simulink model "SdbRxSigProc"  (R2022b) (continued)
== system SdbRxSigProc/SdbRxSigProc_Runnable_sys == (continued)
  DrvrSeatVentnDesPwrCns2_DrvrSeatVentnDesPwrCns2_read/1 -> Terminator_151/1
  DrvrSeatVentnLvlSts2_DrvrSeatVentnLvlSts2_read/1 -> Terminator_152/1
  EngSt1WdSts_EngSt1WdSts_read/1 -> Terminator_153/1
  EngSt1WdSts_EngSt1WdSts_IsUpdated_read/1 -> Terminator_154/1
  FctvSts_FctvSts_read/1 -> Terminator_155/1
  FloatDrvingActv_FloatDrvingActv_read/1 -> Terminator_156/1
  FrntAirPtcHVPwrActl_FrntAirPtcHVPwrActl_read/1 -> Terminator_157/1
  FrntAirPtcHVPwrActl_FrntAirPtcHVPwrActl_IsUpdated_read/1 -> Terminator_158/1
  HighTempCleanHmiReq_HighTempCleanHmiReq_read/1 -> Terminator_159/1
  HmiAC_HmiAC_read/1 -> Terminator_160/1
  HmiAC_HmiAC_IsUpdated_read/1 -> Terminator_161/1
  HMIAirCdnrAutDrySet_HMIAirCdnrAutDrySet_read/1 -> Terminator_162/1
  HMIAirCdnrAutDrySet_HMIAirCdnrAutDrySet_IsUpdated_read/1 -> Terminator_163/1
  HmiAirDistbnRow1_HmiAirDistbnRow1_read/1 -> Terminator_164/1
  HmiAirDistbnRow1_HmiAirDistbnRow1_IsUpdated_read/1 -> Terminator_165/1
  HmiAirDistbnRow1Le_HmiAirDistbnRow1Le_read/1 -> Terminator_166/1
  HmiAirDistbnRow1Ri_HmiAirDistbnRow1Ri_read/1 -> Terminator_167/1
  HmiAirDistbnRow2_HmiAirDistbnRow2_read/1 -> Terminator_168/1
  HmiAirDistbnRow2_HmiAirDistbnRow2_IsUpdated_read/1 -> Terminator_169/1
  HmiAutoRow1_HmiAutoRow1_read/1 -> Terminator_170/1
  HmiAutoRow1_HmiAutoRow1_IsUpdated_read/1 -> Terminator_171/1
  HmiAutoRow1Le_HmiAutoRow1Le_read/1 -> Terminator_172/1
  HmiAutoRow1Ri_HmiAutoRow1Ri_read/1 -> Terminator_173/1
  HmiAutoRow2_HmiAutoRow2_read/1 -> Terminator_174/1
  HmiAutoRow2_HmiAutoRow2_IsUpdated_read/1 -> Terminator_175/1
  HmiAutOxySet_HmiAutOxySet_read/1 -> Terminator_176/1
  HMIBluetoothCallToAdjAirVol_HMIBluetoothCallToAdjAirVol_read/1 -> Terminator_177/1
  HmiCarLockACRecircSet_HmiCarLockACRecircSet_read/1 -> Terminator_178/1
  HmiCbnOverHeatPrevnReq_HmiCbnOverHeatPrevnReq_read/1 -> Terminator_179/1
  HmiCbnOverHeatPrevnReq_HmiCbnOverHeatPrevnReq_IsUpdated_read/1 -> Terminator_180/1
  HmiClimaRow1LePwr_HmiClimaRow1LePwr_read/1 -> Terminator_181/1
  HmiClimaRow1Pwr_HmiClimaRow1Pwr_read/1 -> Terminator_182/1
  HmiClimaRow1RiPwr_HmiClimaRow1RiPwr_read/1 -> Terminator_183/1
  HmiClimaRow2Pwr_HmiClimaRow2Pwr_read/1 -> Terminator_184/1
  HmiClimaRow3Pwr_HmiClimaRow3Pwr_read/1 -> Terminator_185/1
  HMIClimaVentSet_HMIClimaVentSet_read/1 -> Terminator_186/1
  HmiCmptmtAirDistbnFrnt_HmiCmptmtAirDistbnFrnt_read/1 -> Terminator_187/1
  HmiDrvrHeadPosn_HmiDrvrHeadPosn_read/1 -> Terminator_188/1
  HmiEcoClimaReq_HmiEcoClimaReq_read/1 -> Terminator_189/1
  HmiEcoClimaReq_HmiEcoClimaReq_IsUpdated_read/1 -> Terminator_190/1
  HmiElecAirBodyVentLebtn_HmiElecAirBodyVentLebtn_read/1 -> Terminator_191/1
  HmiElecAirBodyVentRibtn_HmiElecAirBodyVentRibtn_read/1 -> Terminator_192/1
  HmiElecAirConsolePosn_HmiElecAirConsolePosn_read/1 -> Terminator_193/1
  HmiElecAirConsoleSwtLe_HmiElecAirConsoleSwtLe_read/1 -> Terminator_194/1
  HmiElecAirConsoleSwtRi_HmiElecAirConsoleSwtRi_read/1 -> Terminator_195/1
  HmiElecAirDirModeSecRowReq_HmiElecAirDirModeSecRowReq_read/1 -> Terminator_196/1
  HmiElecAirDirSwngSecLe_HmiElecAirDirSwngSecLe_read/1 -> Terminator_197/1
  HmiElecAirDirSwngSecRi_HmiElecAirDirSwngSecRi_read/1 -> Terminator_198/1
  HmiElecAirDrvrNatureWindReq_HmiElecAirDrvrNatureWindReq_read/1 -> Terminator_199/1
  HmiElecAirPassNatureWindReq_HmiElecAirPassNatureWindReq_read/1 -> Terminator_200/1
  HMIFACSwt_HMIFACSwt_read/1 -> Terminator_201/1
  HmiFanLvlRow1_HmiFanLvlRow1_read/1 -> Terminator_202/1
  HmiFanLvlRow1_HmiFanLvlRow1_IsUpdated_read/1 -> Terminator_203/1
  HmiFanLvlRow1Le_HmiFanLvlRow1Le_read/1 -> Terminator_204/1
  HmiFanLvlRow1Ri_HmiFanLvlRow1Ri_read/1 -> Terminator_205/1
  HmiFanLvlRow2_HmiFanLvlRow2_read/1 -> Terminator_206/1
  HmiFanLvlRow2_HmiFanLvlRow2_IsUpdated_read/1 -> Terminator_207/1
  HmiFragraCh_HmiFragraCh_read/1 -> Terminator_208/1
  HmiFragraCh_HmiFragraCh_IsUpdated_read/1 -> Terminator_209/1
  HmiFragraLvl_HmiFragraLvl_read/1 -> Terminator_210/1
  HmiFragraLvl_HmiFragraLvl_IsUpdated_read/1 -> Terminator_211/1
  HMIIonizerAirCdnr_HMIIonizerAirCdnr_read/1 -> Terminator_212/1
  HMIIonizerAirCdnr_HMIIonizerAirCdnr_IsUpdated_read/1 -> Terminator_213/1
  HMILBRACStrtSet_HMILBRACStrtSet_read/1 -> Terminator_214/1
  HmiMaxAC_HmiMaxAC_read/1 -> Terminator_215/1
  HmiMaxAC_HmiMaxAC_IsUpdated_read/1 -> Terminator_216/1
  HmiMaxDefrst_HmiMaxDefrst_read/1 -> Terminator_217/1
  HmiMaxDefrst_HmiMaxDefrst_IsUpdated_read/1 -> Terminator_218/1
  HmiOxyinCarSwt_HmiOxyinCarSwt_read/1 -> Terminator_219/1
  HmiParkVentSet_HmiParkVentSet_read/1 -> Terminator_220/1
  HmiParkVentTmrSet_HmiParkVentTmrSet_read/1 -> Terminator_221/1
  HmiPassHeadPosn_HmiPassHeadPosn_read/1 -> Terminator_222/1
  HmiPM25PopUpResp_HmiPM25PopUpResp_read/1 -> Terminator_223/1
  HmiPM25PopUpResp_HmiPM25PopUpResp_IsUpdated_read/1 -> Terminator_224/1
  HmiPM25PopUpSet_HmiPM25PopUpSet_read/1 -> Terminator_225/1
  HmiPM25PopUpSet_HmiPM25PopUpSet_IsUpdated_read/1 -> Terminator_226/1
  HmiSmrtWindFolwSet_HmiSmrtWindFolwSet_read/1 -> Terminator_227/1
  HMIThermoTankDoorOpen_HMIThermoTankDoorOpen_read/1 -> Terminator_228/1
  HMIThermoTankEcoSw_HMIThermoTankEcoSw_read/1 -> Terminator_229/1
  HMIThermoTankT_HMIThermoTankT_read/1 -> Terminator_230/1
  HmiThermoTSteDeoNewSwt_HmiThermoTSteDeoNewSwt_read/1 -> Terminator_231/1
  HmiThermoTSteDeoSwt_HmiThermoTSteDeoSwt_read/1 -> Terminator_232/1
  HmiTSyncn_HmiTSyncn_read/1 -> Terminator_233/1
  HmiTSyncn_HmiTSyncn_IsUpdated_read/1 -> Terminator_234/1
  HmiUVSterilSet_HmiUVSterilSet_read/1 -> Terminator_235/1
  HmiWinterLockCarPrevnIceSet_HmiWinterLockCarPrevnIceSet_read/1 -> Terminator_236/1
  HmiWinterLockCarPrevnIceSet_HmiWinterLockCarPrevnIceSet_IsUpdated_read/1 -> Terminator_237/1
  HoodSts_HoodSts_read/1 -> Terminator_238/1
  HvBattLimnIndcn_HvBattLimnIndcn_read/1 -> Terminator_239/1
  InflateAirSrcSwt_InflateAirSrcSwt_read/1 -> Terminator_240/1
  InpVoltFb_InpVoltFb_read/1 -> Terminator_241/1
  LiOprnMod_LiOprnMod_read/1 -> Terminator_242/1
  LonglifeBatteryReq_LonglifeBatteryReq_read/1 -> Terminator_243/1
  McuOvrTempErr_McuOvrTempErr_read/1 -> Terminator_244/1
  McuTempFb_McuTempFb_read/1 -> Terminator_245/1
  McuTempSnsrErr_McuTempSnsrErr_read/1 -> Terminator_246/1
  MoleculeVlv1_MoleculeVlv1_read/1 -> Terminator_247/1
  MoleculeVlv2_MoleculeVlv2_read/1 -> Terminator_248/1
  MosOvrTempErr_MosOvrTempErr_read/1 -> Terminator_249/1
  MosTempFb_MosTempFb_read/1 -> Terminator_250/1
  MosTempSnsrErr_MosTempSnsrErr_read/1 -> Terminator_251/1
  MotStsFrmOGM_MotStsFrmOGM_read/1 -> Terminator_252/1
  MotTempFb_MotTempFb_read/1 -> Terminator_253/1
  MotTempSnsrErr_MotTempSnsrErr_read/1 -> Terminator_254/1
  OGMCmprSpdFb_OGMCmprSpdFb_read/1 -> Terminator_255/1
  OGMFlowFb_OGMFlowFb_read/1 -> Terminator_256/1
  OGMHwVers_OGMHwVers_read/1 -> Terminator_257/1
  OGMIntErr_OGMIntErr_read/1 -> Terminator_258/1
  OGMOvrVoltRangErr_OGMOvrVoltRangErr_read/1 -> Terminator_259/1
  OGMRunTi_OGMRunTi_read/1 -> Terminator_260/1
  OGMSwVers_OGMSwVers_read/1 -> Terminator_261/1
  OGMTempErr_OGMTempErr_read/1 -> Terminator_262/1
  OGMUndVoltRangErr_OGMUndVoltRangErr_read/1 -> Terminator_263/1
  OxygenConcFbFrmOGM_OxygenConcFbFrmOGM_read/1 -> Terminator_264/1
  OxygenConcFlt_OxygenConcFlt_read/1 -> Terminator_265/1
  OxygenConcSnsrElecErr_OxygenConcSnsrElecErr_read/1 -> Terminator_266/1
  OxygenConcSnsrIntErr_OxygenConcSnsrIntErr_read/1 -> Terminator_267/1
  OxygenConcSnsrOvrVoltRangErr_OxygenConcSnsrOvrVoltRangErr_read/1 -> Terminator_268/1
  OxygenConcSnsrSts_OxygenConcSnsrSts_read/1 -> Terminator_269/1
  OxygenConcSnsrStsFrmOGM_OxygenConcSnsrStsFrmOGM_read/1 -> Terminator_270/1
  OxygenConcSnsrTempErr_OxygenConcSnsrTempErr_read/1 -> Terminator_271/1
  OxygenConcSnsrUndVoltRangErr_OxygenConcSnsrUndVoltRangErr_read/1 -> Terminator_272/1
  OxygenGenrErrInfo_OxygenGenrErrInfo_read/1 -> Terminator_273/1
  OxygenSplyAvlSts_OxygenSplyAvlSts_read/1 -> Terminator_274/1
  OxygenSplyErr_OxygenSplyErr_read/1 -> Terminator_275/1
  OxygenTubeBlk_OxygenTubeBlk_read/1 -> Terminator_276/1
  PassSeatPosPerc_PassSeatPosPerc_read/1 -> Terminator_277/1
  PassSeatPosPerc_PassSeatPosPerc_IsUpdated_read/1 -> Terminator_278/1
  PassSeatSts2_PassSeatSts2_read/1 -> Terminator_279/1
  PassSeatVentnActlPwrCns2_PassSeatVentnActlPwrCns2_read/1 -> Terminator_280/1
  PassSeatVentnDesPwrCns2_PassSeatVentnDesPwrCns2_read/1 -> Terminator_281/1
  PassSeatVentnLvlSts2_PassSeatVentnLvlSts2_read/1 -> Terminator_282/1
  PhaseCurrentFb_PhaseCurrentFb_read/1 -> Terminator_283/1
  PosnBrief_PosnBrief_read/1 -> Terminator_284/1
  PressureFb_PressureFb_read/1 -> Terminator_285/1
  PressureFlt_PressureFlt_read/1 -> Terminator_286/1
  PressureSnsrErr_PressureSnsrErr_read/1 -> Terminator_287/1
  RefrLpSwSts_RefrLpSwSts_read/1 -> Terminator_288/1
  RefrtorVlvEna_RefrtorVlvEna_read/1 -> Terminator_289/1
  ResrvdSigForCCM2_ResrvdSigForCCM2_read/1 -> Terminator_290/1
  RoadIncln_RoadIncln_read/1 -> Terminator_291/1
  RuranACModeSet_RuranACModeSet_read/1 -> Terminator_292/1
  SeatBackAngleRowFirstDrvr_SeatBackAngleRowFirstDrvr_read/1 -> Terminator_293/1
  SeatBackAngleRowFirstDrvr_SeatBackAngleRowFirstDrvr_IsUpdated_read/1 -> Terminator_294/1
  SeatBackAngleRowFirstPass_SeatBackAngleRowFirstPass_read/1 -> Terminator_295/1
  SeatBackAngleRowFirstPass_SeatBackAngleRowFirstPass_IsUpdated_read/1 -> Terminator_296/1
  SeatOccptAtRowSecDrSide2_SeatOccptAtRowSecDrSide2_read/1 -> Terminator_297/1
  SeatOccptAtRowSecMid2_SeatOccptAtRowSecMid2_read/1 -> Terminator_298/1
  SeatOccptAtRowSecPasSide2_SeatOccptAtRowSecPasSide2_read/1 -> Terminator_299/1
  SeatOccptAtRowThrdLe2_SeatOccptAtRowThrdLe2_read/1 -> Terminator_300/1
  SeatOccptAtRowThrdRi2_SeatOccptAtRowThrdRi2_read/1 -> Terminator_301/1
  SportModeThmgtRequest_SportModeThmgtRequest_read/1 -> Terminator_302/1
  TCSCooltFlow_TCSCooltFlow_read/1 -> Terminator_303/1
  TCSCooltT_TCSCooltT_read/1 -> Terminator_304/1
  TcsDoorEleBailReq_TcsDoorEleBailReq_read/1 -> Terminator_305/1
  TcsFanReq_TcsFanReq_read/1 -> Terminator_306/1
  TcsModSwtReq_TcsModSwtReq_read/1 -> Terminator_307/1
  TcsOvrheatdProtnSts_TcsOvrheatdProtnSts_read/1 -> Terminator_308/1
  TcsSemiconReq_TcsSemiconReq_read/1 -> Terminator_309/1
  TcsTmrOverReq_TcsTmrOverReq_read/1 -> Terminator_310/1
  TcsTNowCtrlReq_TcsTNowCtrlReq_read/1 -> Terminator_311/1
  TcsTopDoorEleBailReq_TcsTopDoorEleBailReq_read/1 -> Terminator_312/1
  TelmOxygenValReq_TelmOxygenValReq_read/1 -> Terminator_313/1
  TelmThermoTankECOSet_TelmThermoTankECOSet_read/1 -> Terminator_314/1
  TelmThermoTankT_TelmThermoTankT_read/1 -> Terminator_315/1
  ThermHvacRecircReq_ThermHvacRecircReq_read/1 -> Terminator_316/1
  ThermHvacRecircReqEna_ThermHvacRecircReqEna_read/1 -> Terminator_317/1
  ThrdRowSeatHeatgActlPwrCnsLe_ThrdRowSeatHeatgActlPwrCnsLe_read/1 -> Terminator_318/1
  ThrdRowSeatHeatgActlPwrCnsRi_ThrdRowSeatHeatgActlPwrCnsRi_read/1 -> Terminator_319/1
  ThrdRowSeatHeatgDesPwrCnsLe_ThrdRowSeatHeatgDesPwrCnsLe_read/1 -> Terminator_320/1
  ThrdRowSeatHeatgDesPwrCnsRi_ThrdRowSeatHeatgDesPwrCnsRi_read/1 -> Terminator_321/1
  ThrdRowSeatVentnActlPwrCnsLe_ThrdRowSeatVentnActlPwrCnsLe_read/1 -> Terminator_322/1
  ThrdRowSeatVentnActlPwrCnsRi_ThrdRowSeatVentnActlPwrCnsRi_read/1 -> Terminator_323/1
  ThrdRowSeatVentnDesPwrCnsLe_ThrdRowSeatVentnDesPwrCnsLe_read/1 -> Terminator_324/1
  ThrdRowSeatVentnDesPwrCnsRi_ThrdRowSeatVentnDesPwrCnsRi_read/1 -> Terminator_325/1
  UseEvapReqTMinNew_UseEvapReqTMinNew_read/1 -> Terminator_326/1
  VlvVoltFlt_VlvVoltFlt_read/1 -> Terminator_327/1
  WarnLvlMsg_WarnLvlMsg_read/1 -> Terminator_328/1
  WarnLvlMsg_WarnLvlMsg_IsUpdated_read/1 -> Terminator_329/1
  WasRespLevel_WasRespLevel_read/1 -> Terminator_330/1
  WasRespLevel_WasRespLevel_IsUpdated_read/1 -> Terminator_331/1
  WaterDepthInformation_WaterDepthInformation_read/1 -> Terminator_332/1
  WeatherQualityAQI_WeatherQualityAQI_read/1 -> Terminator_333/1
  Ground_1/1 -> AACAirClngErrStsNgcc_AACAirClngErrStsNgcc_write/1
  Ground_2/1 -> AACAirClngStsNgcc_AACAirClngStsNgcc_write/1
  Ground_3/1 -> AFUFragraCh1AvlTiPrecNgcc_AFUFragraCh1AvlTiPrecNgcc_write/1
  Ground_4/1 -> AFUFragraCh2AvlTiPrecNgcc_AFUFragraCh2AvlTiPrecNgcc_write/1
  Ground_5/1 -> AFUFragraCh3AvlTiPrecNgcc_AFUFragraCh3AvlTiPrecNgcc_write/1
  Ground_6/1 -> AFUFragraCh4AvlTiPrecNgcc_AFUFragraCh4AvlTiPrecNgcc_write/1
  Ground_7/1 -> AFUFragraCh5AvlTiPrecNgcc_AFUFragraCh5AvlTiPrecNgcc_write/1
  Ground_8/1 -> AirClngReq_AirClngReq_write/1
  Ground_9/1(Out1) -> AirFragCh1OvrdDyNgcc_AirFragCh1OvrdDyNgcc_write/1
  Ground_10/1(Out1) -> AirFragCh2OvrdDyNgcc_AirFragCh2OvrdDyNgcc_write/1
  Ground_11/1(Out1) -> AirFragCh3OvrdDyNgcc_AirFragCh3OvrdDyNgcc_write/1
  Ground_12/1(Out1) -> AirFragCh4OvrdDyNgcc_AirFragCh4OvrdDyNgcc_write/1
  Ground_13/1(Out1) -> AirFragCh5OvrdDyNgcc_AirFragCh5OvrdDyNgcc_write/1
  Ground_14/1 -> AirPtcEnadNgcc_AirPtcEnadNgcc_write/1
  Ground_15/1 -> AirPtcHVPwrActlFirstLeNgcc_AirPtcHVPwrActlFirstLeNgcc_write/1
  Ground_16/1 -> AirPtcHVPwrActlFirstRiNgcc_AirPtcHVPwrActlFirstRiNgcc_write/1
  Ground_17/1 -> AirPtcHVPwrActlSecLeNgcc_AirPtcHVPwrActlSecLeNgcc_write/1
  Ground_18/1 -> AirPtcModeReqNgcc_AirPtcModeReqNgcc_write/1
  Ground_19/1 -> AirSrcStsFbNgcc_AirSrcStsFbNgcc_write/1
  Ground_20/1 -> AltitudeInfromNgcc_AltitudeInfromNgcc_write/1
  Ground_21/1(Out1) -> AMBStsNgcc_AMBStsNgcc_write/1
  Ground_22/1 -> BackflushVlvErrNgcc_BackflushVlvErrNgcc_write/1
  Ground_23/1 -> BookTrvlClimaActvReq_Ngcc_BookTrvlClimaActvReq_write/1
  Ground_24/1 -> BusCurrentFbNgcc_BusCurrentFbNgcc_write/1
  Ground_25/1 -> ChgFilElmWarnNgcc_ChgFilElmWarnNgcc_write/1
  Ground_26/1 -> CmptmtOxygenConcQFNgcc_CmptmtOxygenConcQFNgcc_write/1
  Ground_27/1 -> CmptmtOxygenConcVluNgcc_CmptmtOxygenConcVluNgcc_write/1
  Ground_28/1 -> CoolgFanErrNgcc_CoolgFanErrNgcc_write/1
  Ground_29/1(Out1) -> CourseDirFromNavNgcc_CourseDirFromNavNgcc_write/1
  Ground_30/1 -> CurrentCongestionInformationNgcc_CurrentCongestionInformationNgcc_write/1
  Ground_31/1 -> CurrentTunnelInformationNgcc_CurrentTunnelInformationNgcc_write/1
  Ground_32/1 -> DcDcActvdNgcc_DcDcActvdNgcc_write/1
  Ground_33/1 -> DcDcPwrLimNgcc_DcDcPwrLimNgcc_write/1
  Ground_34/1 -> DHUResdSig10Ngcc_DHUResdSig10Ngcc_write/1
  Ground_35/1 -> DHUResdSig11Ngcc_DHUResdSig11Ngcc_write/1
  Ground_36/1 -> DHUResdSig12Ngcc_DHUResdSig12Ngcc_write/1
  Ground_37/1 -> DHUResdSig13Ngcc_DHUResdSig13Ngcc_write/1
  Ground_38/1 -> DHUResdSig3Ngcc_DHUResdSig3Ngcc_write/1
  Ground_39/1 -> DHUResdSig4Ngcc_DHUResdSig4Ngcc_write/1
  Ground_40/1 -> DHUResdSig5Ngcc_DHUResdSig5Ngcc_write/1
  Ground_41/1 -> DHUResdSig6Ngcc_DHUResdSig6Ngcc_write/1
  Ground_42/1 -> DHUResdSig7Ngcc_DHUResdSig7Ngcc_write/1
  Ground_43/1 -> DHUResdSig8Ngcc_DHUResdSig8Ngcc_write/1
  Ground_44/1 -> DHUResdSig9Ngcc_DHUResdSig9Ngcc_write/1
  Ground_45/1 -> DispHvBattLvlOfChrgNgcc_DispHvBattLvlOfChrgNgcc_write/1
  Ground_46/1 -> DropTopPosnStsNgcc_DropTopPosnStsNgcc_write/1
  Ground_47/1(Out1) -> DrvrSeatPosPercNgcc_DrvrSeatPosPercNgcc_write/1
  Ground_48/1 -> DrvrSeatSts_Ngcc_DrvrSeatSts_write/1
  Ground_49/1 -> DrvrSeatVentnLvlSts2Ngcc_DrvrSeatVentnLvlSts2Ngcc_write/1
  Ground_50/1(Out1) -> FctvStsNgcc_FctvStsNgcc_write/1
  Ground_51/1(Out1) -> FloatDrvingActvNgcc_FloatDrvingActvNgcc_write/1
  Ground_52/1 -> HighTempCleanHmiReqNgcc_HighTempCleanHmiReqNgcc_write/1
  Ground_53/1 -> HMIAirCdnrAutDrySetNgcc_HMIAirCdnrAutDrySetNgcc_write/1
  Ground_54/1 -> HmiAirDistbnRow1LeNgcc_HmiAirDistbnRow1LeNgcc_write/1
  Ground_55/1 -> HmiAirDistbnRow1RiNgcc_HmiAirDistbnRow1RiNgcc_write/1
  Ground_56/1 -> HmiAutoRow1LeNgcc_HmiAutoRow1LeNgcc_write/1
  Ground_57/1 -> HmiAutoRow1RiNgcc_HmiAutoRow1RiNgcc_write/1
  Ground_58/1 -> HmiAutOxySetNgcc_HmiAutOxySetNgcc_write/1
  Ground_59/1 -> HmiCarLockACRecircSetNgcc_HmiCarLockACRecircSetNgcc_write/1
  Ground_60/1 -> HmiClimaRow1LePwrNgcc_HmiClimaRow1LePwrNgcc_write/1
  Ground_61/1 -> HmiClimaRow1PwrNgcc_HmiClimaRow1PwrNgcc_write/1
  Ground_62/1 -> HmiClimaRow1RiPwrNgcc_HmiClimaRow1RiPwrNgcc_write/1
  Ground_63/1 -> HmiClimaRow2PwrNgcc_HmiClimaRow2PwrNgcc_write/1
  Ground_64/1 -> HmiClimaRow3PwrNgcc_HmiClimaRow3PwrNgcc_write/1
  Ground_65/1 -> HMIClimaVentSetNgcc_HMIClimaVentSetNgcc_write/1
  Ground_66/1(Out1) -> HmiDrvrHeadPosnNgcc_HmiDrvrHeadPosnNgcc_write/1
  Ground_67/1 -> HmiElecAirBodyVentLebtnNgcc_HmiElecAirBodyVentLebtnNgcc_write/1
  Ground_68/1 -> HmiElecAirBodyVentRibtnNgcc_HmiElecAirBodyVentRibtnNgcc_write/1
  Ground_69/1(Out1) -> HmiElecAirConsolePosnNgcc_HmiElecAirConsolePosnNgcc_write/1
  Ground_70/1 -> HmiElecAirConsoleSwtLeNgcc_HmiElecAirConsoleSwtLeNgcc_write/1
  Ground_71/1 -> HmiElecAirConsoleSwtRiNgcc_HmiElecAirConsoleSwtRiNgcc_write/1
  Ground_72/1(Out1) -> HmiElecAirDirModeSecRowReqNgcc_HmiElecAirDirModeSecRowReqNgcc_write/1
  Ground_73/1 -> HmiElecAirDirSwngSecLeNgcc_HmiElecAirDirSwngSecLeNgcc_write/1
  Ground_74/1 -> HmiElecAirDirSwngSecRiNgcc_HmiElecAirDirSwngSecRiNgcc_write/1
  Ground_75/1 -> HmiElecAirDrvrNatureWindReqNgcc_HmiElecAirDrvrNatureWindReqNgcc_write/1
  Ground_76/1 -> HmiElecAirPassNatureWindReqNgcc_HmiElecAirPassNatureWindReqNgcc_write/1
  Ground_77/1 -> HMIFACSwtNgcc_HMIFACSwtNgcc_write/1
  Ground_78/1 -> HmiFanLvlRow1LeNgcc_HmiFanLvlRow1LeNgcc_write/1
  Ground_79/1 -> HmiFanLvlRow1RiNgcc_HmiFanLvlRow1RiNgcc_write/1
  Ground_80/1(Out1) -> HmiFragraChRatReq_HmiFragraChRatReq_write/1
  Ground_81/1 -> HmiFragraLvlReq_HmiFragraLvlReq_write/1
  Ground_82/1 -> HMILBRACStrtSetNgcc_HMILBRACStrtSetNgcc_write/1
  Ground_83/1 -> HmiOxyinCarSwtNgcc_HmiOxyinCarSwtNgcc_write/1
  Ground_84/1 -> HmiParkVentSetNgcc_HmiParkVentSetNgcc_write/1
  Ground_85/1 -> HmiParkVentTmrSetNgcc_HmiParkVentTmrSetNgcc_write/1
  Ground_86/1(Out1) -> HmiPassHeadPosnNgcc_HmiPassHeadPosnNgcc_write/1
  Ground_87/1 -> HmiPopUpResp_HmiPopUpResp_write/1
  Ground_88/1 -> HmiSmrtWindFolwSetNgcc_HmiSmrtWindFolwSetNgcc_write/1
  Ground_89/1 -> HMIThermoTankDoorOpenNgcc_HMIThermoTankDoorOpenNgcc_write/1
  Ground_90/1 -> HMIThermoTankEcoSwNgcc_HMIThermoTankEcoSwNgcc_write/1
  Ground_91/1 -> HMIThermoTankTNgcc_HMIThermoTankTNgcc_write/1
  Ground_92/1 -> HmiThermoTSteDeoNewSwtNgcc_HmiThermoTSteDeoNewSwtNgcc_write/1
  Ground_93/1 -> HmiThermoTSteDeoSwtNgcc_HmiThermoTSteDeoSwtNgcc_write/1
  Ground_94/1 -> HmiUVSterilSetNgcc_HmiUVSterilSetNgcc_write/1
  Ground_95/1 -> HmiWinterLockCarPrevnIceSetNgcc_HmiWinterLockCarPrevnIceSetNgcc_write/1
  Ground_96/1 -> HoodStsNgcc_HoodStsNgcc_write/1
  Ground_97/1 -> HvBattLimnIndcnNgcc_HvBattLimnIndcnNgcc_write/1
  Ground_98/1 -> InflateAirSrcSwtNgcc_InflateAirSrcSwtNgcc_write/1
  Ground_99/1 -> InpVoltFbNgcc_InpVoltFbNgcc_write/1
  Ground_100/1 -> LiOprnModNgcc_LiOprnModNgcc_write/1
  Ground_101/1 -> LonglifeBatteryReqNgcc_LonglifeBatteryReqNgcc_write/1
  Ground_102/1 -> McuOvrTempErrNgcc_McuOvrTempErrNgcc_write/1
  Ground_103/1 -> McuTempFbNgcc_McuTempFbNgcc_write/1
  Ground_104/1 -> McuTempSnsrErrNgcc_McuTempSnsrErrNgcc_write/1
  Ground_105/1 -> MoleculeVlv1Ngcc_MoleculeVlv1Ngcc_write/1
  Ground_106/1 -> MoleculeVlv2Ngcc_MoleculeVlv2Ngcc_write/1
  Ground_107/1 -> MosOvrTempErrNgcc_MosOvrTempErrNgcc_write/1
  Ground_108/1 -> MosTempFbNgcc_MosTempFbNgcc_write/1
  Ground_109/1 -> MosTempSnsrErrNgcc_MosTempSnsrErrNgcc_write/1
  Ground_110/1 -> MotStsFrmOGMNgcc_MotStsFrmOGMNgcc_write/1
  Ground_111/1 -> MotTempFbNgcc_MotTempFbNgcc_write/1
  Ground_112/1 -> MotTempSnsrErrNgcc_MotTempSnsrErrNgcc_write/1
  Ground_113/1 -> OGMCmprSpdFbNgcc_OGMCmprSpdFbNgcc_write/1
  Ground_114/1 -> OGMFlowFbNgcc_OGMFlowFbNgcc_write/1
  Ground_115/1 -> OGMHwVersNgcc_OGMHwVersNgcc_write/1
  Ground_116/1 -> OGMIntErrNgcc_OGMIntErrNgcc_write/1
  Ground_117/1 -> OGMOvrVoltRangErrNgcc_OGMOvrVoltRangErrNgcc_write/1
  Ground_118/1 -> OGMRunTiNgcc_OGMRunTiNgcc_write/1
  Ground_119/1 -> OGMSwVersNgcc_OGMSwVersNgcc_write/1
  Ground_120/1 -> OGMTempErrNgcc_OGMTempErrNgcc_write/1
  Ground_121/1 -> OGMUndVoltRangErrNgcc_OGMUndVoltRangErrNgcc_write/1
  Ground_122/1 -> OxygenConcFbFrmOGMNgcc_OxygenConcFbFrmOGMNgcc_write/1
  Ground_123/1 -> OxygenConcFltNgcc_OxygenConcFltNgcc_write/1
  Ground_124/1 -> OxygenConcSnsrElecErrNgcc_OxygenConcSnsrElecErrNgcc_write/1
  Ground_125/1 -> OxygenConcSnsrIntErrNgcc_OxygenConcSnsrIntErrNgcc_write/1
  Ground_126/1 -> OxygenConcSnsrOvrVoltRangErrNgcc_OxygenConcSnsrOvrVoltRangErrNgcc_write/1
  Ground_127/1 -> OxygenConcSnsrStsFrmOGMNgcc_OxygenConcSnsrStsFrmOGMNgcc_write/1
  Ground_128/1 -> OxygenConcSnsrStsNgcc_OxygenConcSnsrStsNgcc_write/1
  Ground_129/1 -> OxygenConcSnsrTempErrNgcc_OxygenConcSnsrTempErrNgcc_write/1
  Ground_130/1 -> OxygenConcSnsrUndVoltRangErrNgcc_OxygenConcSnsrUndVoltRangErrNgcc_write/1
  Ground_131/1 -> OxygenGenrErrInfoNgcc_OxygenGenrErrInfoNgcc_write/1
  Ground_132/1 -> OxygenSplyAvlStsNgcc_OxygenSplyAvlStsNgcc_write/1
  Ground_133/1 -> OxygenSplyErrNgcc_OxygenSplyErrNgcc_write/1
  Ground_134/1 -> OxygenTubeBlkNgcc_OxygenTubeBlkNgcc_write/1
  Ground_135/1(Out1) -> PassSeatPosPercNgcc_PassSeatPosPercNgcc_write/1
  Ground_136/1 -> PassSeatSts2_Ngcc_PassSeatSts2_write/1
  Ground_137/1 -> PassSeatVentnLvlSts2Ngcc_PassSeatVentnLvlSts2Ngcc_write/1
  Ground_138/1 -> PhaseCurrentFbNgcc_PhaseCurrentFbNgcc_write/1
  Ground_139/1(Out1) -> PosnBriefNgcc_PosnBriefNgcc_write/1
  Ground_140/1 -> PressureFbNgcc_PressureFbNgcc_write/1
  Ground_141/1 -> PressureFltNgcc_PressureFltNgcc_write/1
  Ground_142/1 -> PressureSnsrErrNgcc_PressureSnsrErrNgcc_write/1
  Ground_143/1 -> PwrCnsForHvacHeatrElecRe_PwrCnsForHvacHeatrElecRe_write/1
  Ground_144/1 -> RefrLpSwStsNgcc_RefrLpSwStsNgcc_write/1
  Ground_145/1 -> RefrtorVlvEnaNgcc_RefrtorVlvEnaNgcc_write/1
  Ground_146/1(Out1) -> RoadInclnNgcc_RoadInclnNgcc_write/1
  Ground_147/1 -> RuranACModeSetNgcc_RuranACModeSetNgcc_write/1
  Ground_148/1 -> SceneModSeld_Ngcc_SceneModSeld_write/1
  Ground_149/1 -> SeatBackAngleRowFirstDrvrNgcc_SeatBackAngleRowFirstDrvrNgcc_write/1
  Ground_150/1 -> SeatBackAngleRowFirstPassNgcc_SeatBackAngleRowFirstPassNgcc_write/1
  Ground_151/1 -> SeatOccptAtRowSecDrSide2_Ngcc_SeatOccptAtRowSecDrSide2_write/1
  Ground_152/1 -> SeatOccptAtRowSecMid2_Ngcc_SeatOccptAtRowSecMid2_write/1
  Ground_153/1 -> SeatOccptAtRowSecPasSide2_Ngcc_SeatOccptAtRowSecPasSide2_write/1
  Ground_154/1 -> SeatOccptAtRowThrdLe2Ngcc_SeatOccptAtRowThrdLe2Ngcc_write/1
  Ground_155/1 -> SeatOccptAtRowThrdRi2Ngcc_SeatOccptAtRowThrdRi2Ngcc_write/1
  Ground_156/1 -> SportModeThmgtRequestNgcc_SportModeThmgtRequestNgcc_write/1
  Ground_157/1 -> TCSCooltFlowNgcc_TCSCooltFlowNgcc_write/1
  Ground_158/1 -> TCSCooltTNgcc_TCSCooltTNgcc_write/1
  Ground_159/1 -> TcsDoorEleBailReqNgcc_TcsDoorEleBailReqNgcc_write/1
  Ground_160/1 -> TcsFanReqNgcc_TcsFanReqNgcc_write/1
  Ground_161/1 -> TcsModSwtReqNgcc_TcsModSwtReqNgcc_write/1
  Ground_162/1 -> TcsOvrheatdProtnStsNgcc_TcsOvrheatdProtnStsNgcc_write/1
  Ground_163/1 -> TcsSemiconReqNgcc_TcsSemiconReqNgcc_write/1
  Ground_164/1 -> TcsTmrOverReqNgcc_TcsTmrOverReqNgcc_write/1
  Ground_165/1 -> TcsTNowCtrlReqNgcc_TcsTNowCtrlReqNgcc_write/1
  Ground_166/1 -> TcsTopDoorEleBailReqNgcc_TcsTopDoorEleBailReqNgcc_write/1
  Ground_167/1 -> TelmOxygenValReqNgcc_TelmOxygenValReqNgcc_write/1
  Ground_168/1 -> TelmThermoTankECOSetNgcc_TelmThermoTankECOSetNgcc_write/1
  Ground_169/1 -> TelmThermoTankTNgcc_TelmThermoTankTNgcc_write/1
  Ground_170/1 -> ThermHvacRecircReqEnaNgcc_ThermHvacRecircReqEnaNgcc_write/1
  Ground_171/1 -> ThermHvacRecircReqNgcc_ThermHvacRecircReqNgcc_write/1
  Ground_172/1 -> ThrdRowSeatHeatgActlPwrCnsLeNgcc_ThrdRowSeatHeatgActlPwrCnsLeNgcc_write/1
  Ground_173/1 -> ThrdRowSeatHeatgActlPwrCnsRiNgcc_ThrdRowSeatHeatgActlPwrCnsRiNgcc_write/1
  Ground_174/1 -> ThrdRowSeatHeatgDesPwrCnsLeNgcc_ThrdRowSeatHeatgDesPwrCnsLeNgcc_write/1
  Ground_175/1 -> ThrdRowSeatHeatgDesPwrCnsRiNgcc_ThrdRowSeatHeatgDesPwrCnsRiNgcc_write/1
  Ground_176/1 -> ThrdRowSeatVentnActlPwrCnsLeNgcc_ThrdRowSeatVentnActlPwrCnsLeNgcc_write/1
  Ground_177/1 -> ThrdRowSeatVentnActlPwrCnsRiNgcc_ThrdRowSeatVentnActlPwrCnsRiNgcc_write/1
  Ground_178/1 -> ThrdRowSeatVentnDesPwrCnsLeNgcc_ThrdRowSeatVentnDesPwrCnsLeNgcc_write/1
  Ground_179/1 -> ThrdRowSeatVentnDesPwrCnsRiNgcc_ThrdRowSeatVentnDesPwrCnsRiNgcc_write/1
  Ground_180/1 -> UseEvapReqTMinNewNgcc_UseEvapReqTMinNewNgcc_write/1
  Ground_181/1(Out1) -> VehTiAndDataNgcc_VehTiAndDataNgcc_write/1
  Ground_182/1 -> VlvVoltFltNgcc_VlvVoltFltNgcc_write/1
  Ground_183/1 -> WarnLvlMsgNgcc_WarnLvlMsgNgcc_write/1
  Ground_184/1 -> WasRespLevelNgcc_WasRespLevelNgcc_write/1
  Ground_185/1(Out1) -> WaterDepthInformationNgcc_WaterDepthInformationNgcc_write/1
  Ground_186/1 -> WeatherQualityAQINgcc_WeatherQualityAQINgcc_write/1
  Ground_187/1 -> WinPosnStsDrv_WinPosnStsAtDrv_write/1
  Ground_188/1 -> ActvnOfWshrFrntSafe_Ngcc_ActvnOfWshrFrntSafe_write/1
  Ground_189/1 -> AirMtrlSnsrInAirQlyIdx_AirMtrlSnsrInAirQlyIdx_write/1
  Ground_190/1 -> AirTAftHeatElmEstimd_AirTAftHeatElmEstimd_write/1
  Ground_191/1 -> AirTFromHeatgEstimd_Ngcc_AirTFromHeatgEstimd_write/1
  Ground_192/1(Out1) -> AmbTOutOfVeh_AmbTOutOfVeh_write/1
  Ground_193/1(Out1) -> AmbTRaw_Ngcc_AmbTRaw_write/1
  Ground_194/1 -> AsyFanSts_AsyFanSts_write/1
  Ground_195/1(Out1) -> BltLockStAtDrvr_Ngcc_BltLockStAtDrvr_write/1
  Ground_196/1(Out1) -> BltLockStAtPass_Ngcc_BltLockStAtPass_write/1
  Ground_197/1(Out1) -> BltLockStAtRowSecLe_Ngcc_BltLockStAtRowSecLe_write/1
  Ground_198/1(Out1) -> BltLockStAtRowSecMid_Ngcc_BltLockStAtRowSecMid_write/1
  Ground_199/1(Out1) -> BltLockStAtRowSecRi_Ngcc_BltLockStAtRowSecRi_write/1
  Ground_200/1(Out1) -> BltLockStAtRowThrdLe_Ngcc_BltLockStAtRowThrdLe_write/1
  Ground_201/1(Out1) -> BltLockStAtRowThrdRi_Ngcc_BltLockStAtRowThrdRi_write/1
  Ground_202/1 -> CamHeatrActv_CamHeatrActv_write/1
  Ground_203/1 -> CarTiGlb_Ngcc_CarTiGlb_write/1
  Ground_204/1 -> ClimaCmd_Ngcc_ClimaCmd_write/1
  Ground_205/1 -> ClimaPwrAllwd_Ngcc_ClimaPwrAllwd_write/1
  Ground_206/1 -> ClimaRqrd1ConnGen2_ClimaRqrd1ConnGen2_write/1
  Ground_207/1 -> ClimaRqrdFromRem1_ClimaRqrdFromRem1_write/1
  Ground_208/1 -> ClimaTmr_ClimaTmr_write/1
  Ground_209/1 -> ClimaTmrStsRqrd_ClimaTmrStsRqrd_write/1
  Ground_210/1 -> ClngRqrdFromHmi_ClngRqrdFromHmi_write/1
  Ground_211/1 -> ClngRqrdFromTelm_ClngRqrdFromTelm_write/1
  Ground_212/1 -> ClngRqrdFromTelmConnGen2_ClngRqrdFromTelmConnGen2_write/1
  Ground_213/1 -> CmpmtInAirPmAQI_Ngcc_CmpmtInAirPmAQI_write/1
  Ground_214/1 -> CmpmtInAirPmDnsty_Ngcc_CmpmtInAirPmDnsty_write/1
  Ground_215/1 -> CmptmtClngPreStrtReqNgcc_CmptmtClngPreStrtReq_write/1
  Ground_216/1 -> CmptmtCoolgSts_Ngcc_CmptmtCoolgSts_write/1
  Ground_217/1(Out1) -> CmptmtRelHum_Ngcc_CmptmtRelHum_write/1
  Ground_218/1(Out1) -> CoolgLoad_CoolgLoad_write/1
  Ground_219/1 -> CoolgReqForDispCen_CoolgReqForDispCen_write/1
  Ground_220/1 -> CoolgReqForDrvrDisp_CoolgReqForDrvrDisp_write/1
  Ground_221/1(Out1) -> CooltHeatrAvlStsForPrkgClima_CooltHeatrAvlStsForPrkgClima_write/1
  Ground_222/1 -> CooltTForPrkgClima_CooltTForPrkgClima_write/1
  Ground_223/1 -> CooltTInHeatElm_CooltTInHeatElm_write/1
  Ground_224/1 -> CooltVlvClsd_CooltVlvClsd_write/1
  Ground_225/1 -> CupHoldrDesPwr_CupHoldrDesPwr_write/1
  Ground_226/1(Out1) -> DefrstSts_Ngcc_DefrstSts_write/1
  Ground_227/1 -> DoorDrvrReSts_DoorDrvrReSts_write/1
  Ground_228/1 -> DoorDrvrSts_Ngcc_DoorDrvrSts_write/1
  Ground_229/1 -> DoorPassReSts_DoorPassReSts_write/1
  Ground_230/1 -> DoorPassSts_Ngcc_DoorPassSts_write/1
  Ground_231/1 -> DrvModReq_Ngcc_DrvModReq_write/1
  Ground_232/1(Out1) -> DrvrAirCdnrSetg_Ngcc_DrvrAirCdnrSetg_write/1
  Ground_233/1 -> DrvrSeatHeatgActlPwrCns_Ngcc_DrvrSeatHeatgActlPwrCns_write/1
  Ground_234/1 -> DrvrSeatHeatgDesPwrCns_Ngcc_DrvrSeatHeatgDesPwrCns_write/1
  Ground_235/1 -> DrvrSeatVentnDesPwrCns_Ngcc_DrvrSeatVentnDesPwrCns_write/1
  Ground_236/1 -> EcuKeepActvFromHeatg_EcuKeepActvFromHeatg_write/1
  Ground_237/1 -> EgyCnsAllwdForClima_Ngcc_EgyCnsAllwdForClima_write/1
  Ground_238/1 -> EgySave_EgySave_write/1
  Ground_239/1 -> EngReqInHybForHeatg_EngReqInHybForHeatg_write/1
  Ground_240/1 -> EngSt1WdSts_Ngcc_EngSt1WdSts_write/1
  Ground_241/1(Out1) -> EngT_Ngcc_EngT_write/1
  Ground_242/1(Out1) -> FogOutOfVeh_FogOutOfVeh_write/1
  Ground_243/1 -> HeatdCupPwrCnsAct_HeatdCupPwrCnsAct_write/1
  Ground_244/1 -> HeatgPwrDmdEstimd_HeatgPwrDmdEstimd_write/1
  Ground_245/1 -> HeatrActvnReq_HeatrActvnReq_write/1
  Ground_246/1 -> HMIClimaEgySaveReq_Ngcc_HMIClimaEgySaveReq_write/1
  Ground_247/1 -> HmiClimaFrntAutReq_HmiClimaFrntAutReq_write/1
  Ground_248/1 -> HmiClimaReAutReq_HmiClimaReAutReq_write/1
  Ground_249/1 -> HmiCmptmtAirDistbnFrntLe_HmiCmptmtAirDistbnFrntLe_write/1
  Ground_250/1 -> HmiCmptmtAirDistbnRe_HmiCmptmtAirDistbnRe_write/1
  Ground_251/1 -> HmiCmptmtCoolgReq_HmiCmptmtCoolgReq_write/1
  Ground_252/1 -> HmiDefrstMaxReq_HmiDefrstMaxReq_write/1
  Ground_253/1 -> HmiHvacFanLvlFrnt_HmiHvacFanLvlFrnt_write/1
  Ground_254/1 -> HmiHvacFanLvlRe_HmiHvacFanLvlRe_write/1
  Ground_255/1 -> HmiMaxACReq_HmiMaxACReq_write/1
  Ground_256/1 -> HmiPassTempSyncSet_HmiPassTempSyncSet_write/1
  Ground_257/1 -> HpcDeiceReq_Ngcc_HpcDeiceReq_write/1
  Ground_258/1(Out1) -> HumOutOfVeh_HumOutOfVeh_write/1
  Ground_259/1 -> HvacFanIFrnt_HvacFanIFrnt_write/1
  Ground_260/1 -> HvacFanIRe_HvacFanIRe_write/1
  Ground_261/1 -> HvacFanMotURe_HvacFanMotURe_write/1
  Ground_262/1 -> HvacFanSpdFrnt_HvacFanSpdFrnt_write/1
  Ground_263/1(Out1) -> HvBattCoolgPwrHeatgMgrThermPred_HvBattCoolgPwrHeatgMgrThermPred_write/1
  Ground_264/1 -> HvPwrEgyPrio_Ngcc_HvPwrEgyPrio_write/1
  Ground_265/1 -> IHUAPSts_Ngcc_IHUAPSts_write/1
  Ground_266/1 -> IncomingAirQlyCtrlFromHmi_Ngcc_IncomingAirQlyCtrlFromHmi_write/1
  Ground_267/1 -> IncomingAirQlyPopUpReq_Ngcc_IncomingAirQlyPopUpReq_write/1
  Ground_268/1 -> InPm25FanErr_Ngcc_InPm25FanErr_write/1
  Ground_269/1 -> InPm25SnsrSts_Ngcc_InPm25SnsrSts_write/1
  Ground_270/1 -> InteCleanUnpleSmell_Ngcc_InteCleanUnpleSmell_write/1
  Ground_271/1 -> IsgMotTInt_IsgMotTInt_write/1
  Ground_272/1 -> LoadForCmprForAirCdn_LoadForCmprForAirCdn_write/1
  Ground_273/1(Out1) -> LockgCenSts_Ngcc_LockgCenSts_write/1
  Ground_274/1 -> MemSaveFlgForCooltHeatrActvnCtrl_MemSaveFlgForCooltHeatrActvnCtrl_write/1
  Ground_275/1 -> MemSaveFlgForCooltHeatrCtrl_MemSaveFlgForCooltHeatrCtrl_write/1
  Ground_276/1 -> MemSaveFlgForFuTInHeatrMdl_MemSaveFlgForFuTInHeatrMdl_write/1
  Ground_277/1 -> MemSaveFlgForHeatgInpMgr_MemSaveFlgForHeatgInpMgr_write/1
  Ground_278/1 -> MemSaveFlgForSnsrMdlCooltT_MemSaveFlgForSnsrMdlCooltT_write/1
  Ground_279/1(Out1) -> MemWrForCooltHeatrActvnCtrl_MemWrForCooltHeatrActvnCtrl_write/1
  Ground_280/1(Out1) -> MemWrForCooltHeatrCtrl_MemWrForCooltHeatrCtrl_write/1
  Ground_281/1(Out1) -> MemWrForFuTInFuHeatrMdl_MemWrForFuTInFuHeatrMdl_write/1
  Ground_282/1(Out1) -> MemWrForHeatgInpMgr_MemWrForHeatgInpMgr_write/1
  Ground_283/1(Out1) -> MemWrForSnsrMdlCooltT_MemWrForSnsrMdlCooltT_write/1
  Ground_284/1 -> NetReqForHeatg_NetReqForHeatg_write/1
  Ground_285/1 -> OnBdChrgrHndlSts_OnBdChrgrHndlSts_write/1
  Ground_286/1 -> PassSeatHeatgActlPwrCns_Ngcc_PassSeatHeatgActlPwrCns_write/1
  Ground_287/1 -> PassSeatHeatgDesPwrCns_Ngcc_PassSeatHeatgDesPwrCns_write/1
  Ground_288/1 -> PassSeatVentnActlPwrCns_Ngcc_PassSeatVentnActlPwrCns_write/1
  Ground_289/1 -> PassSeatVentnDesPwrCns_Ngcc_PassSeatVentnDesPwrCns_write/1
  Ground_290/1 -> SplyUForClimaCtrl_SplyUForClimaCtrl_write/1
  Ground_291/1 -> PostDrvgClimaReq_Ngcc_PostDrvgClimaReq_write/1
  Ground_292/1 -> PrkgHeatgActv_PrkgHeatgActv_write/1
  Ground_293/1 -> PrkgHeatrStrtd_PrkgHeatrStrtd_write/1
  Ground_294/1 -> PwrCnsDesForSeatHeatg_PwrCnsDesForSeatHeatg_write/1
  Ground_295/1 -> PwrCnsDesForSeatVent_PwrCnsDesForSeatVent_write/1
  Ground_296/1 -> PwrCnsDurgEngStopForFuHeatr_PwrCnsDurgEngStopForFuHeatr_write/1
  Ground_297/1 -> PwrCnsDurgEngStopForHvacCooltVlv_PwrCnsDurgEngStopForHvacCooltVlv_write/1
  Ground_298/1 -> PwrCnsDurgEngStopForWtrPmpAux_PwrCnsDurgEngStopForWtrPmpAux_write/1
  Ground_299/1 -> PwrCnsForFuHeatr_PwrCnsForFuHeatr_write/1
  Ground_300/1 -> PwrCnsForHvacCooltVlv_PwrCnsForHvacCooltVlv_write/1
  Ground_301/1 -> PwrCnsForHvacHeatrElecFrnt_PwrCnsForHvacHeatrElecFrnt_write/1
  Ground_302/1 -> PwrCnsForSeatHeatg_PwrCnsForSeatHeatg_write/1
  Ground_303/1 -> PwrCnsForWtrPmpAux_PwrCnsForWtrPmpAux_write/1
  Ground_304/1 -> PwrStsForPrkgHeatrElec_PwrStsForPrkgHeatrElec_write/1
  Ground_305/1 -> RainfallAmnt_Ngcc_RainfallAmnt_write/1
  Ground_306/1(Out1) -> RemStrtHvCtrlReq_Ngcc_RemStrtHvCtrlReq_write/1
  Ground_307/1 -> SeatHeatDurgClimaEnad_SeatHeatDurgClimaEnad_write/1
  Ground_308/1 -> SeatHeatDurgClimaEnadConnGen2_SeatHeatDurgClimaEnadConnGen2_write/1
  Ground_309/1 -> SeatHeatDurgClimaEnadFromTelm_Ngcc_SeatHeatDurgClimaEnadFromTelm_write/1
  Ground_310/1 -> SecRowSeatHeatgActlPwrCnsLe_Ngcc_SecRowSeatHeatgActlPwrCnsLe_write/1
  Ground_311/1 -> SecRowSeatHeatgActlPwrCnsRi_Ngcc_SecRowSeatHeatgActlPwrCnsRi_write/1
  Ground_312/1 -> SecRowSeatHeatgDesPwrCnsLe_Ngcc_SecRowSeatHeatgDesPwrCnsLe_write/1
  Ground_313/1 -> SecRowSeatHeatgDesPwrCnsRi_Ngcc_SecRowSeatHeatgDesPwrCnsRi_write/1
  Ground_314/1 -> SecRowSeatVentnActlPwrCnsLe_Ngcc_SecRowSeatVentnActlPwrCnsLe_write/1
  Ground_315/1 -> SecRowSeatVentnActlPwrCnsRi_Ngcc_SecRowSeatVentnActlPwrCnsRi_write/1
  Ground_316/1 -> SecRowSeatVentnDesPwrCnsLe_Ngcc_SecRowSeatVentnDesPwrCnsLe_write/1
  Ground_317/1 -> SecRowSeatVentnDesPwrCnsRi_Ngcc_SecRowSeatVentnDesPwrCnsRi_write/1
  Ground_318/1 -> SessionActvOutOfVeh_SessionActvOutOfVeh_write/1
  Ground_319/1 -> SteerWhlHeatgDesPwr_Ngcc_SteerWhlHeatgDesPwr_write/1
  Ground_320/1 -> SteerWhlHeatgDurgClimaEnad_SteerWhlHeatgDurgClimaEnad_write/1
  Ground_321/1 -> SteerWhlHeatgDurgClimEandFromTelmConnGen2_SteerWhlHeatgDurgClimEandFromTelmConnGen2_write/1
  Ground_322/1 -> SteerWhlHeatgPwrAct_Ngcc_SteerWhlHeatgPwrAct_write/1
  Ground_323/1 -> StrtRemSts_StrtRemSts1WdStrtRemSts_write/1
  Ground_324/1 -> StrtRemSts1WdStrtRemSts_Ngcc_StrtRemSts1WdStrtRemSts_write/1
  Ground_325/1 -> SunCurtPosnSts_Ngcc_SunCurtPosnSts_write/1
  Ground_326/1(Out1) -> SunData_SunData_write/1
  Ground_327/1(Out1) -> SunLoadOutOfVeh_SunLoadOutOfVeh_write/1
  Ground_328/1 -> SunRoofPosnSts_Ngcc_SunRoofPosnSts_write/1
  Ground_329/1 -> TelmPM25Req_Ngcc_TelmPM25Req_write/1
  Ground_330/1 -> TiDrvgCycPred_TiDrvgCycPred_write/1
  Ground_331/1 -> TrSts_Ngcc_TrSts_write/1
  Ground_332/1(Out1) -> VehModMngtGlbSafe1_Ngcc_VehModMngtGlbSafe1_write/1
  Ground_333/1(Out1) -> VehSpdLgt_Ngcc_VehSpdLgtSafe_write/1
  Ground_334/1 -> WadeProtnSts_Ngcc_WadeProtnSts_write/1
  Ground_335/1 -> WinGlbCmd_Ngcc_WinGlbCmd_write/1
  Ground_336/1 -> WinPosnStsAtDrvrReNgcc_WinPosnStsAtDrvrReNgcc_write/1
  Ground_337/1 -> WinPosnStsAtPassReNgcc_WinPosnStsAtPassRe_write/1
  Ground_338/1 -> WinPosnStsPass_WinPosnStsAtPass_write/1
  Ground_339/1(Out1) -> WipgInfo_Ngcc_WipgInfo_write/1
  Ground_340/1 -> WtrPmpActvnReq_WtrPmpActvnReq_write/1
